simulink model "nonlinear_double_track_model"  (R2024b) (continued)
== system nonlinear_double_track_model/Vehicle Plant Model/D2704 Tire Model == (continued)
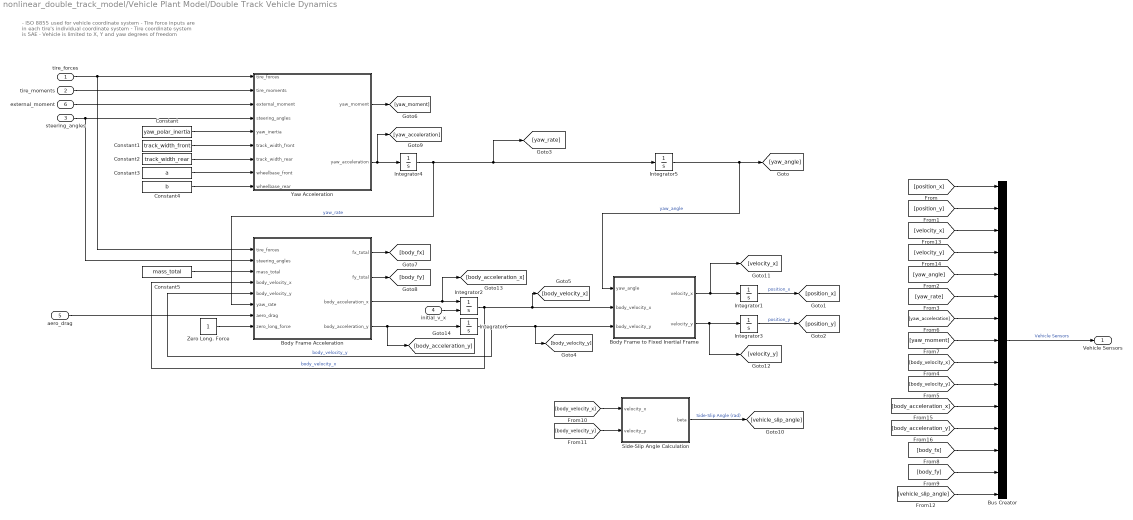
== system nonlinear_double_track_model/Vehicle Plant Model/Double Track Vehicle Dynamics ==
  note: - ISO 8855 used for vehicle coordinate system
      | - Tire force inputs are in each tire's individual coordinate system
      | - Tire coordinate system is SAE
      | - Vehicle is limited to X, Y and yaw degrees of freedom
  tire_forces : Inport
  tire_moments : Inport  Port=2
  steering_angles : Inport  Port=3
  initial_v_x : Inport  Port=4
  aero_drag : Inport  Port=5
  external_moment : Inport  Port=6
  Body Frame Acceleration : MATLAB Function  TreatAsAtomicUnit=on
  Body Frame to Fixed Inertial Frame : MATLAB Function  TreatAsAtomicUnit=on
  Bus Creator : BusCreator  Inputs=15
  Constant : Constant  Value=yaw_polar_inertia
  Constant1 : Constant  Value=track_width_front
  Constant2 : Constant  Value=track_width_rear
  Constant3 : Constant  Value=a
  Constant4 : Constant  Value=b
  Constant5 : Constant  Value=mass_total
  Integrator{1,3..6} (5 alike) : Integrator
  Integrator2 : Integrator  InitialConditionSource=external
  Side-Slip Angle Calculation : MATLAB Function  TreatAsAtomicUnit=on
  Yaw Acceleration : MATLAB Function  TreatAsAtomicUnit=on
  Zero Long. Force : Constant
  Vehicle Sensors : Outport
  wires:
  tire_moments/1 -> Yaw Acceleration/2(tire_moments)
  Bus Creator/1 -> Vehicle Sensors/1  "Vehicle Sensors"
  [position_y] -> Bus Creator/2
  Body Frame Acceleration/4(body_acceleration_y) -> [body_acceleration_y], Integrator6/1
  [position_x] -> Bus Creator/1
  steering_angles/1 -> Body Frame Acceleration/2(steering_angles), Yaw Acceleration/4(steering_angles)
  Integrator2/1 -> Body Frame Acceleration/4(body_velocity_x), [body_velocity_x], Body Frame to Fixed Inertial Frame/2(body_velocity_x)  "body_velocity_x"
  Body Frame to Fixed Inertial Frame/2(velocity_y) -> [velocity_y], Integrator3/1
  Integrator1/1 -> [position_x]  "position_x"
  initial_v_x/1 -> Integrator2/2
  Integrator5/1 -> [yaw_angle], Body Frame to Fixed Inertial Frame/1(yaw_angle)  "yaw_angle"
  Integrator3/1 -> [position_y]  "position_y"
  Body Frame to Fixed Inertial Frame/1(velocity_x) -> [velocity_x], Integrator1/1
  tire_forces/1 -> Body Frame Acceleration/1(tire_forces), Yaw Acceleration/1(tire_forces)
  Integrator4/1 -> Body Frame Acceleration/6(yaw_rate), [yaw_rate], Integrator5/1  "yaw_rate"
  Integrator6/1 -> Body Frame Acceleration/5(body_velocity_y), [body_velocity_y], Body Frame to Fixed Inertial Frame/3(body_velocity_y)  "body_velocity_y"
  Constant/1 -> Yaw Acceleration/5(yaw_inertia)
  Constant4/1 -> Yaw Acceleration/9(wheelbase_rear)
  Constant3/1 -> Yaw Acceleration/8(wheelbase_front)
  Constant2/1 -> Yaw Acceleration/7(track_width_rear)
  Constant1/1 -> Yaw Acceleration/6(track_width_front)
  Yaw Acceleration/2(yaw_acceleration) -> [yaw_acceleration], Integrator4/1
  Body Frame Acceleration/3(body_acceleration_x) -> [body_acceleration_x], Integrator2/1
  Yaw Acceleration/1(yaw_moment) -> [yaw_moment]
  Body Frame Acceleration/1(fx_total) -> [body_fx]
  Body Frame Acceleration/2(fy_total) -> [body_fy]
  external_moment/1 -> Yaw Acceleration/3(external_moment)
  [body_velocity_y] -> Side-Slip Angle Calculation/2(velocity_y)
  [body_velocity_x] -> Side-Slip Angle Calculation/1(velocity_x)
  Side-Slip Angle Calculation/1(beta) -> [vehicle_slip_angle]  "Side-Slip Angle (rad)"
  Zero Long. Force/1 -> Body Frame Acceleration/8(zero_long_force)
  [velocity_y] -> Bus Creator/4
  [velocity_x] -> Bus Creator/3
  [yaw_angle] -> Bus Creator/5
  [yaw_rate] -> Bus Creator/6
  [yaw_acceleration] -> Bus Creator/7
  [yaw_moment] -> Bus Creator/8
  [body_velocity_x] -> Bus Creator/9
  [body_velocity_y] -> Bus Creator/10
  Constant5/1 -> Body Frame Acceleration/3(mass_total)
  aero_drag/1 -> Body Frame Acceleration/7(aero_drag)
  [body_acceleration_y] -> Bus Creator/12
  [body_acceleration_x] -> Bus Creator/11
  [body_fx] -> Bus Creator/13
  [body_fy] -> Bus Creator/14
  [vehicle_slip_angle] -> Bus Creator/15

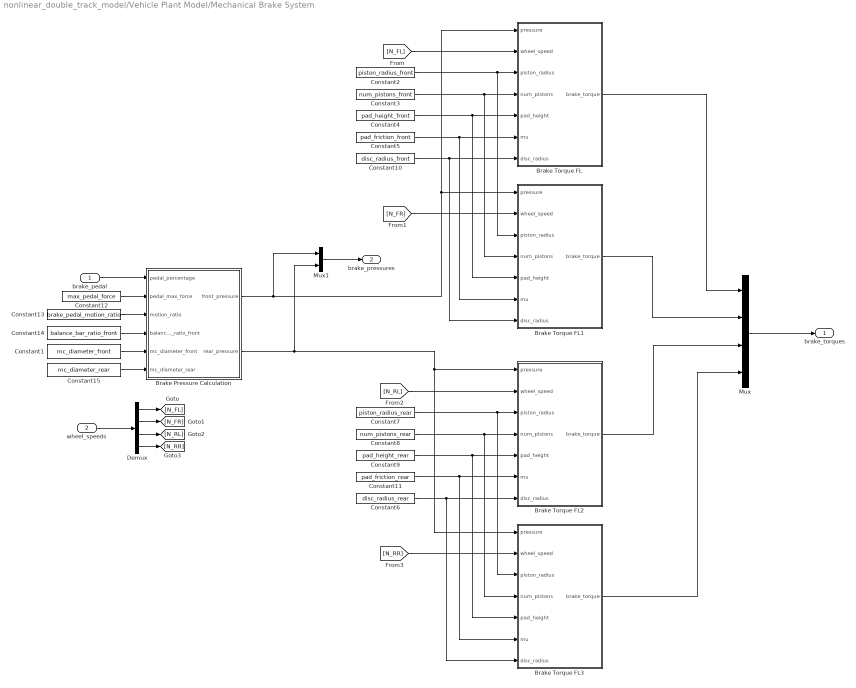
== system nonlinear_double_track_model/Vehicle Plant Model/Mechanical Brake System ==
  brake_pedal : Inport
  wheel_speeds : Inport  Port=2
  Brake Pressure Calculation : MATLAB Function  TreatAsAtomicUnit=on
  Brake Torque FL : MATLAB Function  TreatAsAtomicUnit=on
  Brake Torque FL1 : MATLAB Function  TreatAsAtomicUnit=on
  Brake Torque FL2 : MATLAB Function  TreatAsAtomicUnit=on
  Brake Torque FL3 : MATLAB Function  TreatAsAtomicUnit=on
  Constant1 : Constant  Value=mc_diameter_front
  Constant10 : Constant  Value=disc_radius_front
  Constant11 : Constant  Value=pad_friction_rear
  Constant12 : Constant  Value=max_pedal_force
  Constant13 : Constant  Value=brake_pedal_motion_ratio
  Constant14 : Constant  Value=balance_bar_ratio_front
  Constant15 : Constant  Value=mc_diameter_rear
  Constant2 : Constant  Value=piston_radius_front
  Constant3 : Constant  Value=num_pistons_front
  Constant4 : Constant  Value=pad_height_front
  Constant5 : Constant  Value=pad_friction_front
  Constant6 : Constant  Value=disc_radius_rear
  Constant7 : Constant  Value=piston_radius_rear
  Constant8 : Constant  Value=num_pistons_rear
  Constant9 : Constant  Value=pad_height_rear
  Demux : Demux
  Mux : Mux
  Mux1 : Mux  Inputs=2
  brake_torques : Outport
  brake_pressures : Outport  Port=2
  wires:
  brake_pedal/1 -> Brake Pressure Calculation/1(pedal_percentage)
  Constant2/1 -> Brake Torque FL1/3(piston_radius), Brake Torque FL/3(piston_radius)
  Constant5/1 -> Brake Torque FL1/6(mu), Brake Torque FL/6(mu)
  Constant4/1 -> Brake Torque FL1/5(pad_height), Brake Torque FL/5(pad_height)
  Constant3/1 -> Brake Torque FL1/4(num_pistons), Brake Torque FL/4(num_pistons)
  wheel_speeds/1 -> Demux/1
  Demux/1 -> [N_FL]
  Demux/2 -> [N_FR]
  Demux/3 -> [N_RL]
  Demux/4 -> [N_RR]
  [N_FL] -> Brake Torque FL/2(wheel_speed)
  [N_FR] -> Brake Torque FL1/2(wheel_speed)
  [N_RL] -> Brake Torque FL2/2(wheel_speed)
  [N_RR] -> Brake Torque FL3/2(wheel_speed)
  Brake Pressure Calculation/1(front_pressure) -> Mux1/1, Brake Torque FL1/1(pressure), Brake Torque FL/1(pressure)
  Brake Pressure Calculation/2(rear_pressure) -> Mux1/2, Brake Torque FL3/1(pressure), Brake Torque FL2/1(pressure)
  Mux/1 -> brake_torques/1
  Brake Torque FL1/1(brake_torque) -> Mux/2
  Brake Torque FL2/1(brake_torque) -> Mux/3
  Brake Torque FL3/1(brake_torque) -> Mux/4
  Brake Torque FL/1(brake_torque) -> Mux/1
  Constant10/1 -> Brake Torque FL1/7(disc_radius), Brake Torque FL/7(disc_radius)
  Constant8/1 -> Brake Torque FL3/4(num_pistons), Brake Torque FL2/4(num_pistons)
  Constant11/1 -> Brake Torque FL3/6(mu), Brake Torque FL2/6(mu)
  Constant9/1 -> Brake Torque FL3/5(pad_height), Brake Torque FL2/5(pad_height)
  Constant7/1 -> Brake Torque FL3/3(piston_radius), Brake Torque FL2/3(piston_radius)
  Constant6/1 -> Brake Torque FL3/7(disc_radius), Brake Torque FL2/7(disc_radius)
  Constant12/1 -> Brake Pressure Calculation/2(pedal_max_force)
  Constant13/1 -> Brake Pressure Calculation/3(motion_ratio)
  Constant14/1 -> Brake Pressure Calculation/4(balance_bar_ratio_front)
  Constant1/1 -> Brake Pressure Calculation/5(mc_diameter_front)
  Constant15/1 -> Brake Pressure Calculation/6(mc_diameter_rear)
  Mux1/1 -> brake_pressures/1

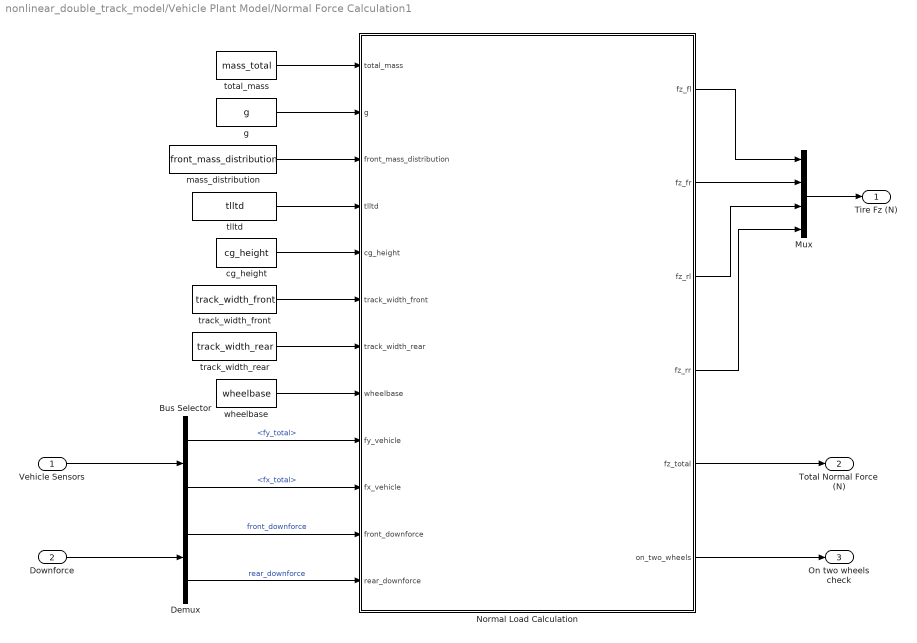
== system nonlinear_double_track_model/Vehicle Plant Model/Normal Force Calculation1 ==
  Vehicle Sensors : Inport
  Downforce : Inport  Port=2
  Bus Selector : BusSelector  OutputSignals=fy_total,fx_total
  Demux : Demux  Outputs=2
  Mux : Mux
  Normal Load Calculation : MATLAB Function  TreatAsAtomicUnit=on
  cg_height : Constant  Value=cg_height
  g : Constant  Value=g
  mass_distribution : Constant  Value=front_mass_distribution
  tlltd : Constant  Value=tlltd
  total_mass : Constant  Value=mass_total
  track_width_front : Constant  Value=track_width_front
  track_width_rear : Constant  Value=track_width_rear
  wheelbase : Constant  Value=wheelbase
  Tire Fz (N) : Outport
  Total Normal Force (N) : Outport  Port=2
  On two wheels check : Outport  Port=3
  wires:
  cg_height/1 -> Normal Load Calculation/5(cg_height)
  Mux/1 -> Tire Fz (N)/1
  total_mass/1 -> Normal Load Calculation/1(total_mass)
  mass_distribution/1 -> Normal Load Calculation/3(front_mass_distribution)
  wheelbase/1 -> Normal Load Calculation/8(wheelbase)
  tlltd/1 -> Normal Load Calculation/4(tlltd)
  track_width_front/1 -> Normal Load Calculation/6(track_width_front)
  track_width_rear/1 -> Normal Load Calculation/7(track_width_rear)
  g/1 -> Normal Load Calculation/2(g)
  Normal Load Calculation/5(fz_total) -> Total Normal Force (N)/1
  Normal Load Calculation/1(fz_fl) -> Mux/1
  Normal Load Calculation/6(on_two_wheels) -> On two wheels check/1
  Normal Load Calculation/2(fz_fr) -> Mux/2
  Normal Load Calculation/3(fz_rl) -> Mux/3
  Normal Load Calculation/4(fz_rr) -> Mux/4
  Vehicle Sensors/1 -> Bus Selector/1
  Bus Selector/2 -> Normal Load Calculation/10(fx_vehicle)  "<fx_total>"
  Bus Selector/1 -> Normal Load Calculation/9(fy_vehicle)  "<fy_total>"
  Downforce/1 -> Demux/1
  Demux/1 -> Normal Load Calculation/11(front_downforce)  "front_downforce"
  Demux/2 -> Normal Load Calculation/12(rear_downforce)  "rear_downforce"

== system nonlinear_double_track_model/Vehicle Plant Model/Slip Angle Calculation ==
  Vehicle Sensors : Inport
  Steering Angles (rad) : Inport  Port=2
  Bus Selector : BusSelector  OutputSignals=body_velocity_y,body_velocity_x,yaw_rate
  Demux : Demux
  Mux : Mux
  Slip Angle Calculation : MATLAB Function  TreatAsAtomicUnit=on
  a : Constant  Value=wheelbase * (1 - front_mass_distribution)
  b : Constant  Value=wheelbase * (front_mass_distribution)
  w : Constant  Value=track_width_rear
  wf : Constant  Value=track_width_front
  Slip Angles (rad) : Outport
  wires:
  a/1 -> Slip Angle Calculation/8(a)
  b/1 -> Slip Angle Calculation/9(b)
  wf/1 -> Slip Angle Calculation/10(tf)
  w/1 -> Slip Angle Calculation/11(tr)
  Slip Angle Calculation/1(alpha_fl) -> Mux/1
  Slip Angle Calculation/2(alpha_fr) -> Mux/2
  Slip Angle Calculation/3(alpha_rl) -> Mux/3
  Slip Angle Calculation/4(alpha_rr) -> Mux/4
  Mux/1 -> Slip Angles (rad)/1
  Demux/1 -> Slip Angle Calculation/4(delta_fl)
  Demux/2 -> Slip Angle Calculation/5(delta_fr)
  Steering Angles (rad)/1 -> Demux/1
  Demux/3 -> Slip Angle Calculation/6(delta_rl)
  Demux/4 -> Slip Angle Calculation/7(delta_rr)
  Vehicle Sensors/1 -> Bus Selector/1
  Bus Selector/2 -> Slip Angle Calculation/2(x_dot)  "<body_velocity_x>"
  Bus Selector/1 -> Slip Angle Calculation/1(y_dot)  "<body_velocity_y>"
  Bus Selector/3 -> Slip Angle Calculation/3(r)  "<yaw_rate>"

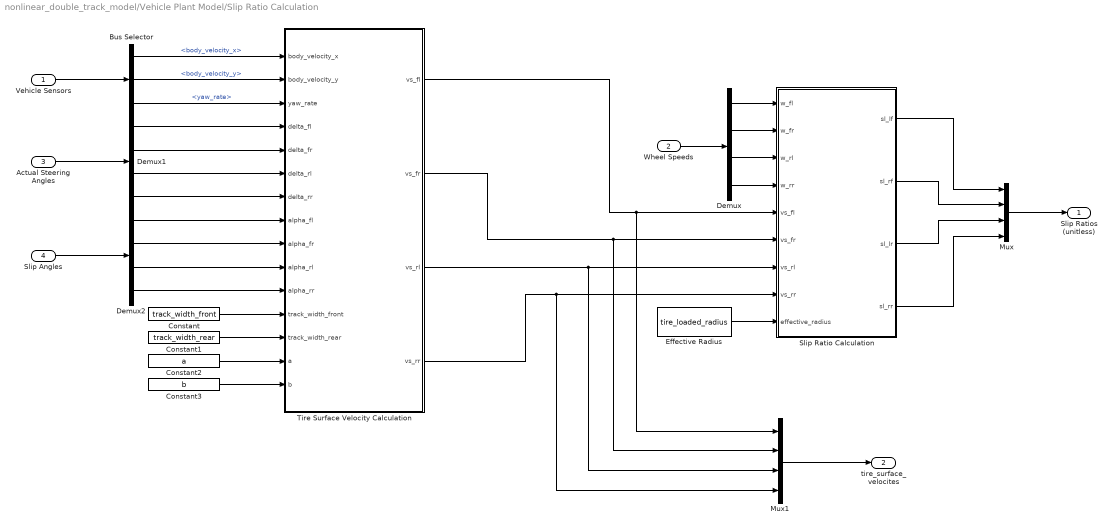
== system nonlinear_double_track_model/Vehicle Plant Model/Slip Ratio Calculation ==
  Vehicle Sensors : Inport
  Wheel Speeds : Inport  Port=2
  Actual Steering Angles : Inport  Port=3
  Slip Angles : Inport  Port=4
  Bus Selector : BusSelector  OutputSignals=body_velocity_x,body_velocity_y,yaw_rate
  Constant : Constant  Value=track_width_front
  Constant1 : Constant  Value=track_width_rear
  Constant2 : Constant  Value=a
  Constant3 : Constant  Value=b
  Demux : Demux
  Demux1 : Demux
  Demux2 : Demux
  Effective Radius : Constant  Value=tire_loaded_radius
  Mux : Mux
  Mux1 : Mux
  Slip Ratio Calculation : MATLAB Function  TreatAsAtomicUnit=on
  Tire Surface Velocity Calculation : MATLAB Function  TreatAsAtomicUnit=on
  Slip Ratios (unitless) : Outport
  tire_surface_velocites : Outport  Port=2
  wires:
  Slip Ratio Calculation/2(sl_rf) -> Mux/2
  Slip Ratio Calculation/4(sl_rr) -> Mux/4
  Slip Ratio Calculation/1(sl_lf) -> Mux/1
  Mux/1 -> Slip Ratios (unitless)/1
  Slip Ratio Calculation/3(sl_lr) -> Mux/3
  Vehicle Sensors/1 -> Bus Selector/1
  Wheel Speeds/1 -> Demux/1
  Bus Selector/2 -> Tire Surface Velocity Calculation/2(body_velocity_y)  "<body_velocity_y>"
  Bus Selector/1 -> Tire Surface Velocity Calculation/1(body_velocity_x)  "<body_velocity_x>"
  Bus Selector/3 -> Tire Surface Velocity Calculation/3(yaw_rate)  "<yaw_rate>"
  Actual Steering Angles/1 -> Demux1/1
  Demux1/1 -> Tire Surface Velocity Calculation/4(delta_fl)
  Demux1/2 -> Tire Surface Velocity Calculation/5(delta_fr)
  Demux1/3 -> Tire Surface Velocity Calculation/6(delta_rl)
  Demux1/4 -> Tire Surface Velocity Calculation/7(delta_rr)
  Demux2/1 -> Tire Surface Velocity Calculation/8(alpha_fl)
  Demux2/2 -> Tire Surface Velocity Calculation/9(alpha_fr)
  Demux2/3 -> Tire Surface Velocity Calculation/10(alpha_rl)
  Demux2/4 -> Tire Surface Velocity Calculation/11(alpha_rr)
  Slip Angles/1 -> Demux2/1
  Constant3/1 -> Tire Surface Velocity Calculation/15(b)
  Constant2/1 -> Tire Surface Velocity Calculation/14(a)
  Constant1/1 -> Tire Surface Velocity Calculation/13(track_width_rear)
  Constant/1 -> Tire Surface Velocity Calculation/12(track_width_front)
  Mux1/1 -> tire_surface_velocites/1
  Demux/4 -> Slip Ratio Calculation/4(w_rr)
  Demux/3 -> Slip Ratio Calculation/3(w_rl)
  Demux/2 -> Slip Ratio Calculation/2(w_fr)
  Demux/1 -> Slip Ratio Calculation/1(w_fl)
  Tire Surface Velocity Calculation/4(vs_rr) -> Slip Ratio Calculation/8(vs_rr), Mux1/4
  Tire Surface Velocity Calculation/3(vs_rl) -> Slip Ratio Calculation/7(vs_rl), Mux1/3
  Tire Surface Velocity Calculation/2(vs_fr) -> Slip Ratio Calculation/6(vs_fr), Mux1/2
  Tire Surface Velocity Calculation/1(vs_fl) -> Slip Ratio Calculation/5(vs_fl), Mux1/1
  Effective Radius/1 -> Slip Ratio Calculation/9(effective_radius)

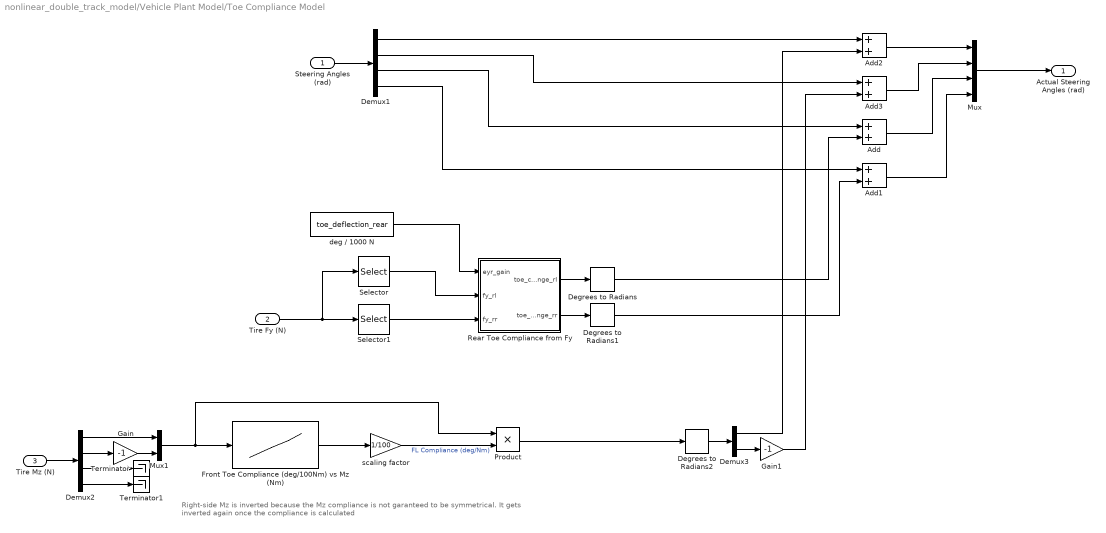
== system nonlinear_double_track_model/Vehicle Plant Model/Toe Compliance Model ==
  note: Right-side Mz is inverted because the Mz compliance is not garanteed to be symmetrical. It gets inverted again once the compliance is calculated
  Steering Angles (rad) : Inport
  Tire Fy (N) : Inport  Port=2
  Tire Mz (N) : Inport  Port=3
  Add{,1..3} (4 alike) : Sum
  Degrees to Radians : simulink_extras/Transformations/Degrees to Radians
  Degrees to Radians1 : simulink_extras/Transformations/Degrees to Radians
  Degrees to Radians2 : simulink_extras/Transformations/Degrees to Radians
  Demux1 : Demux
  Demux2 : Demux
  Demux3 : Demux  Outputs=2
  Front Toe Compliance (deg/100Nm) vs Mz (Nm) : Lookup_n-D  NumberOfTableDimensions=1  BreakpointsForDimension1=[-99.514563;-90.776699;-80.582524;-70.38835;-60.194175;-50.485437;-40.291262;-30.582524;-20.873786;-10.194175;-0.4854369;9.22330097;19.9029126;29.1262136;39.8058252;49.5145631;59.223301;69.9029126;79.6116505;89.3203883;100]  Table=[-2.0208845;-1.9103194;-1.7997543;-1.6769042;-1.529484;-1.3820639;-1.1977887;-0.9889435;-0.7555283;-0.4238329;-0.0061425;0.42383292;0.74324324;1.0012285;1.22235872;1.39434889;1.55405405;1.68918919;1.7997543;1.92260442;2.03316953]  RndMeth=Simplest
  Gain : Gain  Gain=-1
  Gain1 : Gain  Gain=-1
  Mux : Mux
  Mux1 : Mux  Inputs=2
  Product : Product
  Rear Toe Compliance from Fy : MATLAB Function  TreatAsAtomicUnit=on
  Selector : Selector  NumberOfDimensions=2  InputPortWidth=3  IndexOptions=Starting index (dialog),Starting index (dialog)  Indices=1,3  OutputSizes=1,1
  Selector1 : Selector  NumberOfDimensions=2  InputPortWidth=3  IndexOptions=Starting index (dialog),Starting index (dialog)  Indices=1,4  OutputSizes=1,1
  Terminator : Terminator
  Terminator1 : Terminator
  deg / 1000 N : Constant  Value=toe_deflection_rear
  scaling factor : Gain  Gain=1/100
  Actual Steering Angles (rad) : Outport
  wires:
  Tire Fy (N)/1 -> Selector1/1, Selector/1
  deg / 1000 N/1 -> Rear Toe Compliance from Fy/1(eyr_gain)
  Rear Toe Compliance from Fy/1(toe_change_rl) -> Degrees to Radians/1
  Rear Toe Compliance from Fy/2(toe_change_rr) -> Degrees to Radians1/1
  Demux1/3 -> Add/1
  Demux1/4 -> Add1/1
  Degrees to Radians/1 -> Add/2
  Degrees to Radians1/1 -> Add1/2
  Mux/1 -> Actual Steering Angles (rad)/1
  Add/1 -> Mux/3
  Add1/1 -> Mux/4
  Tire Mz (N)/1 -> Demux2/1
  Front Toe Compliance (deg/100Nm) vs Mz (Nm)/1 -> scaling factor/1
  Add2/1 -> Mux/1
  Add3/1 -> Mux/2
  Demux1/1 -> Add2/1
  Demux1/2 -> Add3/1
  Steering Angles (rad)/1 -> Demux1/1
  scaling factor/1 -> Product/2  "FL Compliance (deg/Nm)"
  Demux2/3 -> Terminator/1
  Demux2/4 -> Terminator1/1
  Demux2/1 -> Mux1/1
  Demux2/2 -> Gain/1
  Mux1/1 -> Product/1, Front Toe Compliance (deg/100Nm) vs Mz (Nm)/1
  Degrees to Radians2/1 -> Demux3/1
  Demux3/1 -> Add2/2
  Demux3/2 -> Gain1/1
  Product/1 -> Degrees to Radians2/1
  Gain/1 -> Mux1/2
  Gain1/1 -> Add3/2
  Selector/1 -> Rear Toe Compliance from Fy/2(fy_rl)
  Selector1/1 -> Rear Toe Compliance from Fy/3(fy_rr)

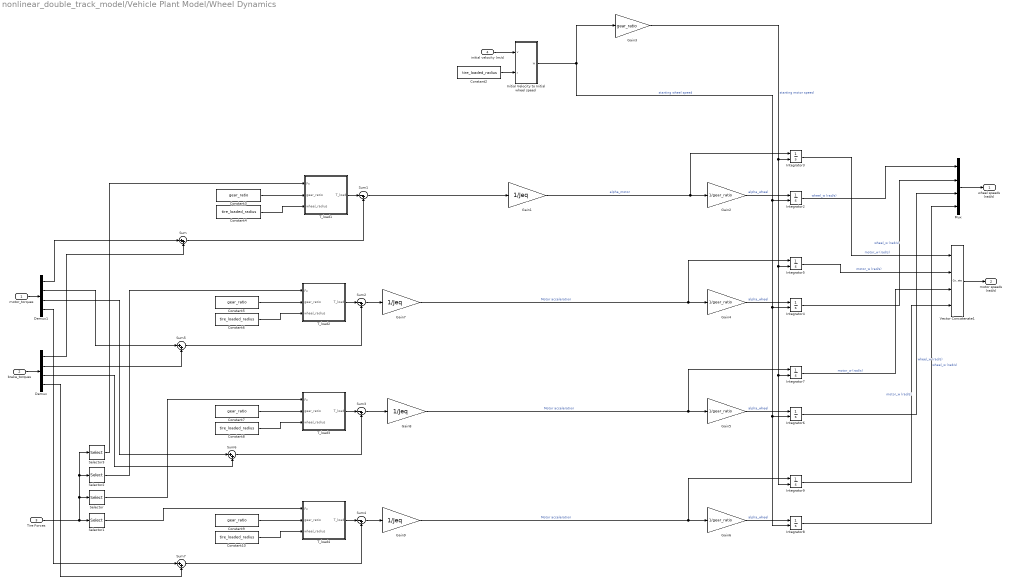
== system nonlinear_double_track_model/Vehicle Plant Model/Wheel Dynamics ==
  motor_torques : Inport
  brake_torques : Inport  Port=2
  Tire Forces : Inport  Port=3
  initial velocity (m/s) : Inport  Port=4
  Constant{2,4,6,8,10} (5 alike) : Constant  Value=tire_loaded_radius
  Constant{3,5,7,9} (4 alike) : Constant  Value=gear_ratio
  Demux : Demux
  Demux1 : Demux
  Gain{1,7..9} (4 alike) : Gain  Gain=1/Jeq
  Gain{2,4..6} (4 alike) : Gain  Gain=1/gear_ratio
  Gain3 : Gain  Gain=gear_ratio
  Initial Velocity to Initial wheel speed : MATLAB Function  TreatAsAtomicUnit=on
  Integrator{2..9} (8 alike) : Integrator  InitialConditionSource=external
  Mux : Mux
  Selector : Selector  NumberOfDimensions=2  InputPortWidth=3  IndexOptions=Starting index (dialog),Starting index (dialog)  Indices=2,3  OutputSizes=1,1
  Selector1 : Selector  NumberOfDimensions=2  InputPortWidth=3  IndexOptions=Starting index (dialog),Starting index (dialog)  Indices=2,4  OutputSizes=1,1
  Selector2 : Selector  NumberOfDimensions=2  InputPortWidth=3  IndexOptions=Starting index (dialog),Starting index (dialog)  Indices=2,2  OutputSizes=1,1
  Selector3 : Selector  NumberOfDimensions=2  InputPortWidth=3  IndexOptions=Starting index (dialog),Starting index (dialog)  Indices=2,1  OutputSizes=1,1
  Sum{,5..7} (4 alike) : Sum  Inputs=|++
  Sum{1..4} (4 alike) : Sum  Inputs=|-+
  T_load1 : MATLAB Function  TreatAsAtomicUnit=on
  T_load2 : MATLAB Function  TreatAsAtomicUnit=on
  T_load3 : MATLAB Function  TreatAsAtomicUnit=on
  T_load4 : MATLAB Function  TreatAsAtomicUnit=on
  Vector Concatenate1 : Concatenate  NumInputs=4  Mode=Multidimensional array  ConcatenateDimension=2
  wheel speeds (rad/s) : Outport
  motor speeds (rad/s) : Outport  Port=2
  wires:
  Gain2/1 -> Integrator2/1  "alpha_wheel"
  initial velocity (m/s)/1 -> Initial Velocity to Initial wheel speed/1(v)
  Constant2/1 -> Initial Velocity to Initial wheel speed/2(r)
  Initial Velocity to Initial wheel speed/1(w) -> Integrator8/2, Integrator6/2, Integrator4/2, Integrator2/2, Gain3/1  "starting wheel speed"
  Gain3/1 -> Integrator9/2, Integrator7/2, Integrator5/2, Integrator3/2  "starting motor speed"
  Constant4/1 -> T_load1/3(wheel_radius)
  Sum1/1 -> Gain1/1
  Selector3/1 -> T_load1/1(Fx)
  Constant3/1 -> T_load1/2(gear_ratio)
  T_load1/1(T_load) -> Sum1/1
  Sum/1 -> Sum1/2
  Constant6/1 -> T_load2/3(wheel_radius)
  Sum2/1 -> Gain7/1
  Gain4/1 -> Integrator4/1  "alpha_wheel"
  Gain7/1 -> Integrator5/1, Gain4/1  "Motor acceleration"
  Selector2/1 -> T_load2/1(Fx)
  T_load2/1(T_load) -> Sum2/1
  Constant5/1 -> T_load2/2(gear_ratio)
  Constant8/1 -> T_load3/3(wheel_radius)
  Sum3/1 -> Gain8/1
  Gain5/1 -> Integrator6/1  "alpha_wheel"
  Gain8/1 -> Integrator7/1, Gain5/1  "Motor acceleration"
  Selector/1 -> T_load3/1(Fx)
  T_load3/1(T_load) -> Sum3/1
  Constant7/1 -> T_load3/2(gear_ratio)
  Constant10/1 -> T_load4/3(wheel_radius)
  Sum4/1 -> Gain9/1
  Gain6/1 -> Integrator8/1  "alpha_wheel"
  Gain9/1 -> Integrator9/1, Gain6/1  "Motor acceleration"
  Selector1/1 -> T_load4/1(Fx)
  T_load4/1(T_load) -> Sum4/1
  Constant9/1 -> T_load4/2(gear_ratio)
  Tire Forces/1 -> Selector1/1, Selector3/1, Selector2/1, Selector/1
  Integrator2/1 -> Mux/1  "wheel_w (rad/s)"
  Integrator4/1 -> Mux/2  "wheel_w (rad/s)"
  Mux/1 -> wheel speeds (rad/s)/1
  Vector Concatenate1/1 -> motor speeds (rad/s)/1
  Integrator6/1 -> Mux/3  "wheel_w (rad/s)"
  Integrator8/1 -> Mux/4  "wheel_w (rad/s)"
  Integrator9/1 -> Vector Concatenate1/4  "motor_w (rad/s)"
  Integrator7/1 -> Vector Concatenate1/3  "motor_w (rad/s)"
  Integrator5/1 -> Vector Concatenate1/2  "motor_w (rad/s)"
  Integrator3/1 -> Vector Concatenate1/1  "motor_w (rad/s)"
  brake_torques/1 -> Demux/1
  Sum5/1 -> Sum2/2
  Sum6/1 -> Sum3/2
  Sum7/1 -> Sum4/2
  motor_torques/1 -> Demux1/1
  Demux1/1 -> Sum/1
  Demux/1 -> Sum/2
  Demux1/2 -> Sum5/1
  Demux/2 -> Sum5/2
  Demux1/3 -> Sum6/1
  Demux1/4 -> Sum7/1
  Demux/3 -> Sum6/2
  Demux/4 -> Sum7/2
  Gain1/1 -> Integrator3/1, Gain2/1  "alpha_motor"

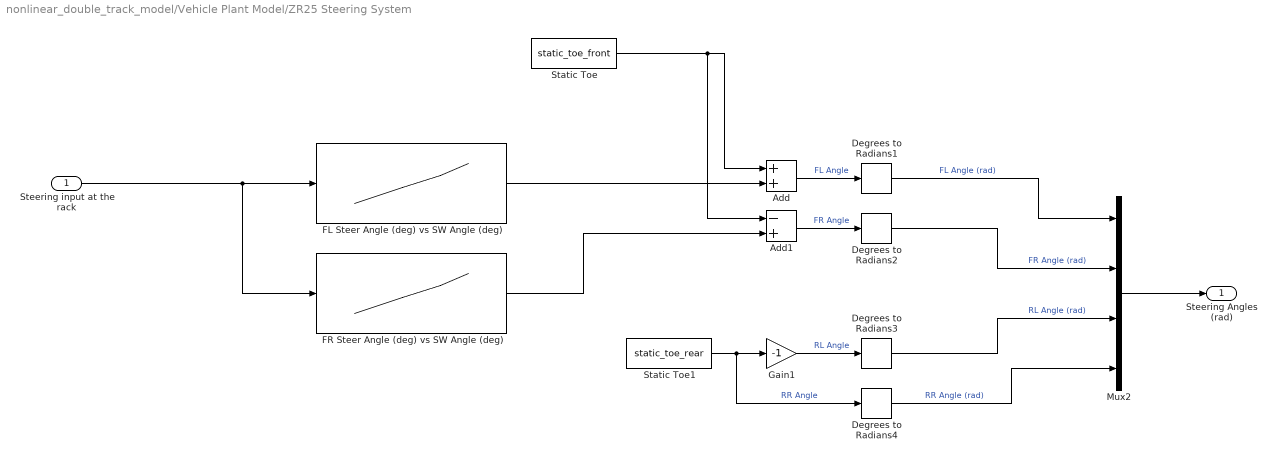
== system nonlinear_double_track_model/Vehicle Plant Model/ZR25 Steering System ==
  Steering input at the rack : Inport
  Add : Sum
  Add1 : Sum  Inputs=-+
  Degrees to Radians{1..4} (4 alike) : simulink_extras/Transformations/Degrees to Radians
  FL Steer Angle (deg) vs SW Angle (deg) : Lookup_n-D  NumberOfTableDimensions=1  BreakpointsForDimension1=[-180;-162;-144;-126;-108;-90;-72;-54;-36;-18;0;18;36;54;72;90;108;126;144;162;180]  Table=[-37.683;-33.549;-29.57;-25.708;-21.934;-18.224;-14.558;-10.918;-7.289;-3.654;0;3.689;7.428;11.234;15.126;19.127;23.261;27.563;32.073;36.847;41.964]  InternalRulePriority=Speed  RndMeth=Simplest
  FR Steer Angle (deg) vs SW Angle (deg) : Lookup_n-D  NumberOfTableDimensions=1  BreakpointsForDimension1=[-180;-162;-144;-126;-108;-90;-72;-54;-36;-18;0;18;36;54;72;90;108;126;144;162;180]  Table=[-41.964;-36.847;-32.073;-27.563;-23.261;-19.126;-15.126;-11.234;-7.428;-3.689;0;3.654;7.289;10.918;14.558;18.225;21.934;25.708;29.57;33.549;37.683]  InternalRulePriority=Speed  RndMeth=Simplest
  Gain1 : Gain  Gain=-1
  Mux2 : Mux
  Static Toe : Constant  Value=static_toe_front
  Static Toe1 : Constant  Value=static_toe_rear
  Steering Angles (rad) : Outport
  wires:
  Steering input at the rack/1 -> FR Steer Angle (deg) vs SW Angle (deg)/1, FL Steer Angle (deg) vs SW Angle (deg)/1
  Add1/1 -> Degrees to Radians2/1  "FR Angle"
  Add/1 -> Degrees to Radians1/1  "FL Angle"
  Static Toe/1 -> Add/1, Add1/1
  Static Toe1/1 -> Gain1/1, Degrees to Radians4/1  "RR Angle"
  Gain1/1 -> Degrees to Radians3/1  "RL Angle"
  Degrees to Radians1/1 -> Mux2/1  "FL Angle (rad)"
  Degrees to Radians2/1 -> Mux2/2  "FR Angle (rad)"
  Degrees to Radians3/1 -> Mux2/3  "RL Angle (rad)"
  Degrees to Radians4/1 -> Mux2/4  "RR Angle (rad)"
  Mux2/1 -> Steering Angles (rad)/1
  FL Steer Angle (deg) vs SW Angle (deg)/1 -> Add/2
  FR Steer Angle (deg) vs SW Angle (deg)/1 -> Add1/2
